simulink library "inp"  (Simulink 8.2)
settings: Solver=ode45  SolverMode=SingleTasking  StartTime=0.0  StopTime=10.0  RelTol=1e-3
undefined symbols (not defined in the file or in its folder): C_TICK_TIME_S, ENUM_FAULTSTATE_FAIL, ENUM_FAULTSTATE_INDETERMINATE, ENUM_FAULTSTATE_PASS, ENUM_KEYSWITCH_ON, FALSE, KINP_ABS1CANCommuOk_flg_ovrdflg, KINP_ABS1CANCommuOk_flg_ovrdval, KINP_ABS1CANPeriodTime_s, KINP_ABS1CANRolCntOk_flg_ovrdflg, KINP_ABS1CANRolCntOk_flg_ovrdval, KINP_ABS2CANChkSumOk_flg_ovrdflg, KINP_ABS2CANChkSumOk_flg_ovrdval, KINP_ABS2CANCommuOk_flg_ovrdflg, KINP_ABS2CANCommuOk_flg_ovrdval, KINP_ABS2CANPeriodTime_s, KINP_ABS2CANRolCntOk_flg_ovrdflg, KINP_ABS2CANRolCntOk_flg_ovrdval, KINP_ABS3CANCommuOk_flg_ovrdflg, KINP_ABS3CANCommuOk_flg_ovrdval, KINP_ABS4CANCommuOk_flg_ovrdflg, KINP_ABS4CANCommuOk_flg_ovrdval, KINP_ABSActive_flg_defval, KINP_ABSActive_flg_invalid, KINP_ABSActive_flg_ovrdflg, KINP_ABSActive_flg_ovrdval, KINP_ABSActive_flg_setvalid, KINP_ABSActive_flg_srvcflg, KINP_ABSActive_flg_srvcval, KINP_ABSCANAlive_flg_ovrdflg, KINP_ABSCANAlive_flg_ovrdval, KINP_ABSCANCommuOk_flg_ovrdflg, KINP_ABSCANCommuOk_flg_ovrdval, KINP_ABSCANValidOk_flg_ovrdflg, KINP_ABSCANValidOk_flg_ovrdval, KINP_ABSFault_flg_defval, KINP_ABSFault_flg_invalid, KINP_ABSFault_flg_ovrdflg, KINP_ABSFault_flg_ovrdval, KINP_ABSFault_flg_setvalid, KINP_ABSFault_flg_srvcflg, KINP_ABSFault_flg_srvcval, KINP_AC1CANCommuOk_flg_ovrdflg, KINP_AC1CANCommuOk_flg_ovrdval, KINP_ACCANAlive_flg_ovrdflg, KINP_ACCANAlive_flg_ovrdval, KINP_ACCANCommuOk_flg_ovrdflg, KINP_ACCANCommuOk_flg_ovrdval, KINP_ACCANValidOk_flg_ovrdflg, KINP_ACCANValidOk_flg_ovrdval, KINP_ACCCancelH_flg_defval, KINP_ACCCancelH_flg_invalid, KINP_ACCCancelH_flg_ovrdflg, KINP_ACCCancelH_flg_ovrdval, KINP_ACCCancelH_flg_setvalid, KINP_ACCCancelH_flg_srvcflg, KINP_ACCCancelH_flg_srvcval, KINP_AccelPedalPosn_pct_defval, KINP_AccelPedalPosn_pct_gain, KINP_AccelPedalPosn_pct_invalid ... (+1866)
libraries: inp x192, hcu_lib x34, simulink x33
(part 5 of 21)

== system inp/INP/METER_signals ==
  BHAL_outputs : Inport  OutDataTypeStr=Bus: BHAL_outputs
  CANvalid_bus : Inport  Port=2
  Bus Selector1 : BusSelector  OutputSignals=VHAL_MeterVehSpd_kph,VHAL_MeterVehSpdInvalid_flg
  Bus Selector10 : BusSelector  OutputSignals=VHAL_SeatBeltNOTFasten_flg
  Bus Selector12 : BusSelector  OutputSignals=VHAL_LowBrakeFluid_flg
  Bus Selector2 : BusSelector  OutputSignals=VINP_CANMsgValidMETER3_flg
  Bus Selector{4,6,9,22} (4 alike) : BusSelector  OutputSignals=VINP_CANMsgValidMETER1_flg
  Bus Selector5 : BusSelector  OutputSignals=VHAL_ParkBrakeOn_flg
  Bus Selector8 : BusSelector  OutputSignals=VHAL_OdoMeter_km,VHAL_OdoMeterInvalid_flg
  Data Store Read1 : DataStoreRead  DataStoreName=VDDML_METER3CANCommuFault_AF_flg
  Data Store Read{7..9,13} (4 alike) : DataStoreRead  DataStoreName=VDDML_METER1CANCommuFault_AF_flg
  Data Store Read14 : DataStoreRead  DataStoreName=VDDML_CANOdoMeterSigFault_AF_flg
  Data Store Read2 : DataStoreRead  DataStoreName=VDDML_CANMeterVehSpdSigFault_AF_flg
  Logical Operator1 : Logic  Tag=struct('inputs','1','comment',struct('text','','placement',1),'logdata',struct('maxnumvals',inf,'plotchannels',[],'stepsize',0,'downsampling',1,'loggingmode',3,'channels',1),'operator','NOT','output',struct('class','default','type','Bool8','description','','address','','unit','','name','$S_$B'),'date',731430.46749816)  Operator=NOT
  Logical Operator14 : Logic  Tag=struct('inputs','1','comment',struct('text','','placement',1),'logdata',struct('maxnumvals',inf,'plotchannels',[],'stepsize',0,'downsampling',1,'loggingmode',3,'channels',1),'operator','NOT','output',struct('class','default','type','Bool8','description','','address','','unit','','name','$S_$B'),'date',731430.46749816)  Operator=NOT
  Logical Operator2 : Logic  Tag=struct('inputs','1','comment',struct('text','','placement',1),'logdata',struct('maxnumvals',inf,'plotchannels',[],'stepsize',0,'downsampling',1,'loggingmode',3,'channels',1),'operator','NOT','output',struct('class','default','type','Bool8','description','','address','','unit','','name','$S_$B'),'date',731430.46749816)  Operator=OR
  Logical Operator6 : Logic  Tag=struct('inputs','1','comment',struct('text','','placement',1),'logdata',struct('maxnumvals',inf,'plotchannels',[],'stepsize',0,'downsampling',1,'loggingmode',3,'channels',1),'operator','NOT','output',struct('class','default','type','Bool8','description','','address','','unit','','name','$S_$B'),'date',731430.46749816)  Operator=OR
  LowBrakeFluid : Subsystem
      settings: RTWSystemCode=Inline
  MeterVehSpd : Subsystem
      settings: RTWSystemCode=Inline
  OdoMeter : Subsystem
      settings: RTWSystemCode=Inline
  ParkBrakeOn : Subsystem
      settings: RTWSystemCode=Inline
  SeatBeltNOTFasten : Subsystem
      settings: RTWSystemCode=Inline
  inputs_bus2 : BusCreator  Inputs=12
  METER_signal_bus : Outport
  wires:
  [BHAL_outputs] -> Bus Selector12/1
  LowBrakeFluid/2(signal_valid_out) -> inputs_bus2/5  "VINP_LowBrakeFluidValid_flg_InHAL"
  Bus Selector12/1 -> LowBrakeFluid/1(signal_in)  "<VHAL_LowBrakeFluid_flg>"
  LowBrakeFluid/1(signal_out) -> inputs_bus2/4  "VINP_LowBrakeFluid_flg_InHAL"
  Logical Operator6/1 -> OdoMeter/4(Active_fault_flg)
  Bus Selector9/1 -> OdoMeter/3(msg_valid)  "<VINP_CANMsgValidMETER1_flg>"
  [CANvalid_bus] -> Bus Selector9/1
  OdoMeter/3(raw_signal_valid_out) -> inputs_bus2/3  "VINP_OdoMeterSigOk_flg_InHAL"
  OdoMeter/2(signal_valid_out) -> inputs_bus2/2  "VINP_OdoMeterValid_flg_InHAL"
  OdoMeter/1(signal_out) -> inputs_bus2/1  "VINP_OdoMeter_km_InHAL"
  Data Store Read13/1 -> Logical Operator6/2
  Data Store Read14/1 -> Logical Operator6/1
  Logical Operator14/1 -> OdoMeter/2(signal_valid_in)
  Bus Selector8/2 -> Logical Operator14/1  "<VHAL_OdoMeterInvalid_flg>"
  Bus Selector8/1 -> OdoMeter/1(signal_in)  "<VHAL_OdoMeter_km>"
  [BHAL_outputs] -> Bus Selector8/1
  CANvalid_bus/1 -> [CANvalid_bus]
  BHAL_outputs/1 -> [BHAL_outputs]
  inputs_bus2/1 -> METER_signal_bus/1
  Bus Selector4/1 -> LowBrakeFluid/2(msg_valid)  "<VINP_CANMsgValidMETER1_flg>"
  [CANvalid_bus] -> Bus Selector4/1
  Data Store Read7/1 -> LowBrakeFluid/3(Active_fault_flg)
  [BHAL_outputs] -> Bus Selector5/1
  ParkBrakeOn/2(signal_valid_out) -> inputs_bus2/7  "VINP_ParkBrakeOnValid_flg_InHAL"
  Bus Selector5/1 -> ParkBrakeOn/1(signal_in)  "<VHAL_ParkBrakeOn_flg>"
  ParkBrakeOn/1(signal_out) -> inputs_bus2/6  "VINP_ParkBrakeOn_flg_InHAL"
  Bus Selector6/1 -> ParkBrakeOn/2(msg_valid)  "<VINP_CANMsgValidMETER1_flg>"
  [CANvalid_bus] -> Bus Selector6/1
  Data Store Read8/1 -> ParkBrakeOn/3(Active_fault_flg)
  [BHAL_outputs] -> Bus Selector10/1
  SeatBeltNOTFasten/2(signal_valid_out) -> inputs_bus2/9  "VINP_SeatBeltNOTFastenValid_flg_InHAL"
  Bus Selector10/1 -> SeatBeltNOTFasten/1(signal_in)  "<VHAL_SeatBeltNOTFasten_flg>"
  SeatBeltNOTFasten/1(signal_out) -> inputs_bus2/8  "VINP_SeatBeltNOTFasten_flg_InHAL"
  Bus Selector22/1 -> SeatBeltNOTFasten/2(msg_valid)  "<VINP_CANMsgValidMETER1_flg>"
  [CANvalid_bus] -> Bus Selector22/1
  Data Store Read9/1 -> SeatBeltNOTFasten/3(Active_fault_flg)
  Logical Operator2/1 -> MeterVehSpd/4(Active_fault_flg)
  Bus Selector2/1 -> MeterVehSpd/3(msg_valid)  "<VINP_CANMsgValidMETER3_flg>"
  [CANvalid_bus] -> Bus Selector2/1
  MeterVehSpd/3(raw_signal_valid_out) -> inputs_bus2/12  "VINP_MeterVehSpdSigOk_flg_InHAL"
  MeterVehSpd/2(signal_valid_out) -> inputs_bus2/11  "VINP_MeterVehSpdValid_flg_InHAL"
  MeterVehSpd/1(signal_out) -> inputs_bus2/10  "VINP_MeterVehSpd_kph_InHAL"
  Data Store Read1/1 -> Logical Operator2/2
  Data Store Read2/1 -> Logical Operator2/1
  Logical Operator1/1 -> MeterVehSpd/2(signal_valid_in)
  Bus Selector1/2 -> Logical Operator1/1  "<VHAL_MeterVehSpdInvalid_flg>"
  Bus Selector1/1 -> MeterVehSpd/1(signal_in)  "<VHAL_MeterVehSpd_kph>"
  [BHAL_outputs] -> Bus Selector1/1

== system inp/INP/TCM_signals ==
  BHAL_outputs : Inport  OutDataTypeStr=Bus: BHAL_outputs
  CANvalid_bus : Inport  Port=2
  Bus Selector{1..2,5,10..11,13,16,31} (8 alike) : BusSelector  OutputSignals=VINP_CANMsgValidTCM1_flg
  Bus Selector12 : BusSelector  OutputSignals=VHAL_TCMMILReq_flg
  Bus Selector14 : BusSelector  OutputSignals=VHAL_TCMFaultStatus_enum
  Bus Selector15 : BusSelector  OutputSignals=VHAL_TCMWinterMode_flg
  Bus Selector17 : BusSelector  OutputSignals=VHAL_TCMPowerMode_flg
  Bus Selector3 : BusSelector  OutputSignals=VHAL_TMTurbineSpd_rpm,VHAL_TMTurbineSpdInvalid_flg
  Bus Selector4 : BusSelector  OutputSignals=VHAL_ShifterPosn_enum,VHAL_ShifterPosnInvalid_flg
  Bus Selector6 : BusSelector  OutputSignals=VHAL_NowGearPosn_enum,VHAL_NowGearPosnInvalid_flg
  Bus Selector7 : BusSelector  OutputSignals=VHAL_DriverMode_enum
  Bus Selector8 : BusSelector  OutputSignals=VINP_CANMsgValidTCM2_flg
  Bus Selector9 : BusSelector  OutputSignals=VHAL_TqConvClutchSt_enum
  Constant{,1..3} (4 alike) : Constant  Value=TRUE
  Data Store Read{1..4,20..21,23,25} (8 alike) : DataStoreRead  DataStoreName=VDDML_TCM1CANCommuFault_AF_flg
  Data Store Read18 : DataStoreRead  DataStoreName=VDDML_CANNowGearPosnSigFault_AF_flg
  Data Store Read19 : DataStoreRead  DataStoreName=VDDML_CANShftPosnSigFault_AF_flg
  Data Store Read24 : DataStoreRead  DataStoreName=VDDML_CANTMTurbSpdSigFault_AF_flg
  Data Store Read32 : DataStoreRead  DataStoreName=VDDML_TCM2CANCommuFault_AF_flg
  Data Type Conversion : DataTypeConversion  OutDataTypeStr=uint8
  Data Type Conversion1 : DataTypeConversion  OutDataTypeStr=boolean
  Data Type Conversion2 : DataTypeConversion  OutDataTypeStr=boolean
  Data Type Conversion3 : DataTypeConversion  OutDataTypeStr=boolean
  DriverMode : Subsystem
      settings: RTWSystemCode=Inline
  Logical Operator1 : Logic  Tag=struct('inputs','1','comment',struct('text','','placement',1),'logdata',struct('maxnumvals',inf,'plotchannels',[],'stepsize',0,'downsampling',1,'loggingmode',3,'channels',1),'operator','NOT','output',struct('class','default','type','Bool8','description','','address','','unit','','name','$S_$B'),'date',731430.46749816)  Operator=OR
  Logical Operator2 : Logic  Tag=struct('inputs','1','comment',struct('text','','placement',1),'logdata',struct('maxnumvals',inf,'plotchannels',[],'stepsize',0,'downsampling',1,'loggingmode',3,'channels',1),'operator','NOT','output',struct('class','default','type','Bool8','description','','address','','unit','','name','$S_$B'),'date',731430.46749816)  Operator=OR
  Logical Operator3 : Logic  Tag=struct('inputs','1','comment',struct('text','','placement',1),'logdata',struct('maxnumvals',inf,'plotchannels',[],'stepsize',0,'downsampling',1,'loggingmode',3,'channels',1),'operator','NOT','output',struct('class','default','type','Bool8','description','','address','','unit','','name','$S_$B'),'date',731430.46749816)  Operator=NOT  Inputs=1
  Logical Operator4 : Logic  Tag=struct('inputs','1','comment',struct('text','','placement',1),'logdata',struct('maxnumvals',inf,'plotchannels',[],'stepsize',0,'downsampling',1,'loggingmode',3,'channels',1),'operator','NOT','output',struct('class','default','type','Bool8','description','','address','','unit','','name','$S_$B'),'date',731430.46749816)  Operator=OR
  Logical Operator5 : Logic  Tag=struct('inputs','1','comment',struct('text','','placement',1),'logdata',struct('maxnumvals',inf,'plotchannels',[],'stepsize',0,'downsampling',1,'loggingmode',3,'channels',1),'operator','NOT','output',struct('class','default','type','Bool8','description','','address','','unit','','name','$S_$B'),'date',731430.46749816)  Operator=NOT  Inputs=1
  Logical Operator8 : Logic  Tag=struct('inputs','1','comment',struct('text','','placement',1),'logdata',struct('maxnumvals',inf,'plotchannels',[],'stepsize',0,'downsampling',1,'loggingmode',3,'channels',1),'operator','NOT','output',struct('class','default','type','Bool8','description','','address','','unit','','name','$S_$B'),'date',731430.46749816)  Operator=NOT  Inputs=1
  NowGearPosn : Subsystem
      settings: RTWSystemCode=Inline
  ShifterPosn : Subsystem
      settings: RTWSystemCode=Inline
  TCMFaultStatus : Subsystem
      settings: RTWSystemCode=Inline
  TMTurbineSpd : Subsystem
      settings: RTWSystemCode=Inline
  Terminator{,1..7} (8 alike) : Terminator
  inputs_bus2 : BusCreator  Inputs=21
  TCM_signal_bus : Outport
  wires:
  inputs_bus2/1 -> TCM_signal_bus/1
  BHAL_outputs/1 -> [BHAL_outputs]
  CANvalid_bus/1 -> [CANvalid_bus]
  Logical Operator8/1 -> ShifterPosn/2(signal_valid_in)
  Bus Selector4/1 -> ShifterPosn/1(signal_in)  "<VHAL_ShifterPosn_enum>"
  [BHAL_outputs] -> Bus Selector4/1
  ShifterPosn/2(signal_valid_out) -> inputs_bus2/2  "VINP_ShifterPosnValid_flg_InHAL"
  ShifterPosn/1(signal_out) -> inputs_bus2/1  "VINP_ShifterPosn_enum_InHAL"
  Bus Selector4/2 -> Logical Operator8/1  "<VHAL_ShifterPosnInvalid_flg>"
  Data Store Read19/1 -> Logical Operator2/1
  [CANvalid_bus] -> Bus Selector1/1
  Bus Selector1/1 -> ShifterPosn/3(msg_valid)  "<VINP_CANMsgValidTCM1_flg>"
  Logical Operator2/1 -> ShifterPosn/4(Active_fault_flg)
  Data Store Read20/1 -> Logical Operator2/2
  ShifterPosn/3(raw_signal_valid_out) -> inputs_bus2/3  "VINP_ShifterPosnSigOk_flg_InHAL"
  Logical Operator3/1 -> NowGearPosn/2(signal_valid_in)
  Bus Selector6/1 -> NowGearPosn/1(signal_in)  "<VHAL_NowGearPosn_enum>"
  [BHAL_outputs] -> Bus Selector6/1
  NowGearPosn/2(signal_valid_out) -> inputs_bus2/5  "VINP_NowGearPosnValid_flg_InHAL"
  NowGearPosn/1(signal_out) -> inputs_bus2/4  "VINP_NowGearPosn_enum_InHAL"
  Bus Selector6/2 -> Logical Operator3/1  "<VHAL_NowGearPosnInvalid_flg>"
  Data Store Read18/1 -> Logical Operator1/1
  [CANvalid_bus] -> Bus Selector5/1
  Bus Selector5/1 -> NowGearPosn/3(msg_valid)  "<VINP_CANMsgValidTCM1_flg>"
  Logical Operator1/1 -> NowGearPosn/4(Active_fault_flg)
  Data Store Read21/1 -> Logical Operator1/2
  NowGearPosn/3(raw_signal_valid_out) -> inputs_bus2/6  "VINP_NowGearPosnSigOk_flg_InHAL"
  TMTurbineSpd/1(signal_out) -> inputs_bus2/7  "VINP_TMTurbineSpd_rpm_InHAL"
  TMTurbineSpd/2(signal_valid_out) -> inputs_bus2/8  "VINP_TMTurbineSpdValid_flg_InHAL"
  TMTurbineSpd/3(raw_signal_valid_out) -> inputs_bus2/9  "VINP_TMTurbineSpdSigOk_flg_InHAL"
  Logical Operator5/1 -> TMTurbineSpd/2(signal_valid_in)
  Bus Selector3/1 -> TMTurbineSpd/1(signal_in)  "<VHAL_TMTurbineSpd_rpm>"
  [BHAL_outputs] -> Bus Selector3/1
  Bus Selector3/2 -> Logical Operator5/1  "<VHAL_TMTurbineSpdInvalid_flg>"
  Data Store Read24/1 -> Logical Operator4/1
  [CANvalid_bus] -> Bus Selector2/1
  Bus Selector2/1 -> TMTurbineSpd/3(msg_valid)  "<VINP_CANMsgValidTCM1_flg>"
  Logical Operator4/1 -> TMTurbineSpd/4(Active_fault_flg)
  Data Store Read25/1 -> Logical Operator4/2
  Bus Selector14/1 -> TCMFaultStatus/1(signal_in)  "<VHAL_TCMFaultStatus_enum>"
  [BHAL_outputs] -> Bus Selector14/1
  TCMFaultStatus/1(signal_out) -> inputs_bus2/10  "VINP_TCMFaultStatus_enum_InHAL"
  TCMFaultStatus/2(signal_valid_out) -> inputs_bus2/11  "VINP_TCMFaultStatusValid_flg_InHAL"
  Bus Selector31/1 -> TCMFaultStatus/2(msg_valid)  "<VINP_CANMsgValidTCM1_flg>"
  [CANvalid_bus] -> Bus Selector31/1
  Data Store Read23/1 -> TCMFaultStatus/3(Active_fault_flg)
  Bus Selector7/1 -> DriverMode/1(signal_in)  "<VHAL_DriverMode_enum>"
  [BHAL_outputs] -> Bus Selector7/1
  DriverMode/1(signal_out) -> inputs_bus2/20  "VINP_DriverMode_enum_InHAL"
  DriverMode/2(signal_valid_out) -> inputs_bus2/21  "VINP_DriverModeValid_flg_InHAL"
  Bus Selector8/1 -> DriverMode/2(msg_valid)  "<VINP_CANMsgValidTCM2_flg>"
  [CANvalid_bus] -> Bus Selector8/1
  Data Store Read32/1 -> DriverMode/3(Active_fault_flg)
  Bus Selector9/1 -> Data Type Conversion/1  "<VHAL_TqConvClutchSt_enum>"
  [BHAL_outputs] -> Bus Selector9/1
  Data Type Conversion/1 -> inputs_bus2/12  "VINP_TqConvClutchSt_enum_InHAL"
  Constant/1 -> inputs_bus2/13  "VINP_TqConvClutchStValid_flg_InHAL"
  Data Store Read2/1 -> Terminator1/1
  Bus Selector10/1 -> Terminator/1  "<VINP_CANMsgValidTCM1_flg>"
  [CANvalid_bus] -> Bus Selector10/1
  Bus Selector12/1 -> Data Type Conversion1/1  "<VHAL_TCMMILReq_flg>"
  [BHAL_outputs] -> Bus Selector12/1
  Data Type Conversion1/1 -> inputs_bus2/14  "VINP_TCMMILReq_flg_InHAL"
  Constant1/1 -> inputs_bus2/15  "VINP_TCMMILReqValid_flg_InHAL"
  Data Store Read1/1 -> Terminator3/1
  Bus Selector11/1 -> Terminator2/1  "<VINP_CANMsgValidTCM1_flg>"
  [CANvalid_bus] -> Bus Selector11/1
  Bus Selector15/1 -> Data Type Conversion2/1  "<VHAL_TCMWinterMode_flg>"
  [BHAL_outputs] -> Bus Selector15/1
  Data Type Conversion2/1 -> inputs_bus2/16  "VINP_TCMWinterMode_flg_InHAL"
  Constant2/1 -> inputs_bus2/17  "VINP_TCMWinterModeValid_flg_InHAL"
  Data Store Read3/1 -> Terminator5/1
  Bus Selector13/1 -> Terminator4/1  "<VINP_CANMsgValidTCM1_flg>"
  [CANvalid_bus] -> Bus Selector13/1
  Bus Selector17/1 -> Data Type Conversion3/1  "<VHAL_TCMPowerMode_flg>"
  [BHAL_outputs] -> Bus Selector17/1
  Data Type Conversion3/1 -> inputs_bus2/18  "VINP_TCMPowerMode_flg_InHAL"
  Constant3/1 -> inputs_bus2/19  "VINP_TCMPowerModeValid_flg_InHAL"
  Data Store Read4/1 -> Terminator7/1
  Bus Selector16/1 -> Terminator6/1  "<VINP_CANMsgValidTCM1_flg>"
  [CANvalid_bus] -> Bus Selector16/1

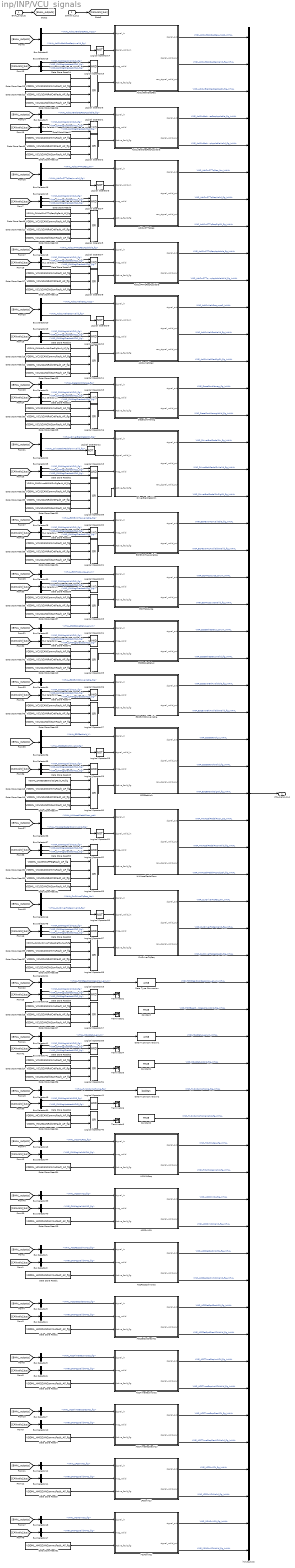
== system inp/INP/VCU_signals ==
  BHAL_outputs : Inport  OutDataTypeStr=Bus: BHAL_outputs
  CANvalid_bus : Inport  Port=2
  ACCOnVCU : Subsystem
      settings: RTWSystemCode=Inline
  ACCResSpdIncVCU : Subsystem
      settings: RTWSystemCode=Inline
  ACCSetSpdDecVCU : Subsystem
      settings: RTWSystemCode=Inline
  ACCTimeGapDecVCU : Subsystem
      settings: RTWSystemCode=Inline
  ACCTimeGapIncVCU : Subsystem
      settings: RTWSystemCode=Inline
  AckCurBrakDecReq : Subsystem
      settings: RTWSystemCode=Inline
  AckCurBrakDecReqApplable : Subsystem
      settings: RTWSystemCode=Inline
  AckCurPTTqReq : Subsystem
      settings: RTWSystemCode=Inline
  AckCurPTTqReqApplable : Subsystem
      settings: RTWSystemCode=Inline
  AckCurVehDec : Subsystem
      settings: RTWSystemCode=Inline
  Bus Selector1 : BusSelector  OutputSignals=VHAL_ACCResSpdIncVCU_flg
  Bus Selector{2,4,6,10..11,40,47} (7 alike) : BusSelector  OutputSignals=VINP_CANMsgValidHMI2_flg
  Bus Selector12 : BusSelector  OutputSignals=VHAL_LFCOnVCU_flg
  Bus Selector{13..15,17,19,23,25,27,29,31,33,35} (12 alike) : BusSelector  OutputSignals=VINP_CANMsgValidVCU1_flg,VINP_CANMsgUpdatedVCU1_flg,VINP_CANMsgCheckedVCU1_flg
  Bus Selector16 : BusSelector  OutputSignals=VHAL_AckCurPTTqReq_Nm,VHAL_AckCurPTTqReqInvalid_flg
  Bus Selector18 : BusSelector  OutputSignals=VHAL_AckCurPTTqReqApplable_flg
  Bus Selector20 : BusSelector  OutputSignals=VHAL_AckCurVehDec_mps2,VHAL_AckCurVehDecInvalid_flg
  Bus Selector21 : BusSelector  OutputSignals=VHAL_TCMGearPosnReqAllow_enum
  Bus Selector22 : BusSelector  OutputSignals=VHAL_DriverBrakPedalOn_flg,VHAL_DriverBrakPedalOnInvalid_flg
  Bus Selector24 : BusSelector  OutputSignals=VHAL_DeceContManag_flg
  Bus Selector{26,37,42,45} (4 alike) : BusSelector  OutputSignals=VINP_CANMsgValidVCU2_flg,VINP_CANMsgUpdatedVCU2_flg,VINP_CANMsgCheckedVCU2_flg
  Bus Selector28 : BusSelector  OutputSignals=VHAL_ECMExtnTqAvailable_flg
  Bus Selector3 : BusSelector  OutputSignals=VHAL_ACCSetSpdDecVCU_flg
  Bus Selector30 : BusSelector  OutputSignals=VHAL_ECMTqSource_enum
  Bus Selector32 : BusSelector  OutputSignals=VHAL_ESCBrakeStatus_enum
  Bus Selector34 : BusSelector  OutputSignals=VHAL_ESCExtnBrkAvailable_flg
  Bus Selector36 : BusSelector  OutputSignals=VHAL_ESCBattVolt_V,VHAL_ESCBattVoltInvalid_flg
  Bus Selector38 : BusSelector  OutputSignals=VHAL_HWAccelPedalPosn_pct,VHAL_HWAccelPedalPosnInvalid_flg
  Bus Selector39 : BusSelector  OutputSignals=VHAL_ACCOnVCU_flg
  Bus Selector41 : BusSelector  OutputSignals=VHAL_MSAStatus_enum
  Bus Selector43 : BusSelector  OutputSignals=VHAL_HCUMILReq_flg
  Bus Selector44 : BusSelector  OutputSignals=VINP_CANMsgValidHCU4_flg
  Bus Selector46 : BusSelector  OutputSignals=VHAL_CurDriverTqReq_Nm,VHAL_CurDriverTqReqInvalid_flg
  Bus Selector48 : BusSelector  OutputSignals=VHAL_MSAOnVCU_flg
  Bus Selector49 : BusSelector  OutputSignals=VHAL_HybridLimpHome_flg
  Bus Selector5 : BusSelector  OutputSignals=VHAL_ACCTimeGapIncVCU_flg
  Bus Selector50 : BusSelector  OutputSignals=VINP_CANMsgValidHCU3_flg,VINP_CANMsgUpdatedHCU3_flg
  Bus Selector7 : BusSelector  OutputSignals=VHAL_ACCTimeGapDecVCU_flg
  Bus Selector8 : BusSelector  OutputSignals=VHAL_AckCurBrakDecReq_mps2,VHAL_AckCurBrakDecReqInvalid_flg
  Bus Selector9 : BusSelector  OutputSignals=VHAL_AckCurBrakDecReqApplable_flg
  Constant : Constant  Value=TRUE
  Constant1 : Constant  Value=TRUE
  Constant2 : Constant  Value=TRUE
  CurDriverTqReq : Subsystem
      settings: RTWSystemCode=Inline
  Data Store Read{1..5,19,61} (7 alike) : DataStoreRead  DataStoreName=VDDML_HMI2CANCommuFault_AF_flg
  Data Store Read{10,12,15,23,26,28,31,34,37,40,43,47} (12 alike) : DataStoreRead  DataStoreName=VDDML_VCU1CANChkSumFault_AF_flg
  Data Store Read{9,11,17,21..22,30,33,36,39,42,45..46} (12 alike) : DataStoreRead  DataStoreName=VDDML_VCU1CANRolCntFault_AF_flg
  Data Store Read{6..7,13,16,18,29,32,35,38,41,44,48} (12 alike) : DataStoreRead  DataStoreName=VDDML_VCU1CANCommuFault_AF_flg
  Data Store Read14 : DataStoreRead  DataStoreName=VDDML_CANAckCurBrakDecReqSigFault_AF_flg
  Data Store Read20 : DataStoreRead  DataStoreName=VDDML_CANAckCurVehDecSigFault_AF_flg
  Data Store Read{24..25,50,57} (4 alike) : DataStoreRead  DataStoreName=VDDML_VCU2CANRolCntFault_AF_flg
  Data Store Read{27,51,56,58} (4 alike) : DataStoreRead  DataStoreName=VDDML_VCU2CANChkSumFault_AF_flg
  Data Store Read49 : DataStoreRead  DataStoreName=VDDML_CANESCBattVoltSigFault_AF_flg
  Data Store Read{52,55,59,63} (4 alike) : DataStoreRead  DataStoreName=VDDML_VCU2CANCommuFault_AF_flg
  Data Store Read53 : DataStoreRead  DataStoreName=VDDML_CANHWAPPSigFault_AF_flg
  Data Store Read54 : DataStoreRead  DataStoreName=VDDML_HCU4CANCommuFault_AF_flg
  Data Store Read60 : DataStoreRead  DataStoreName=VDDML_CANCurDriverTqReqSigFault_AF_flg
  Data Store Read62 : DataStoreRead  DataStoreName=VDDML_CANDriverBrkPdlOnSigFault_AF_flg
  Data Store Read64 : DataStoreRead  DataStoreName=VDDML_HCU3CANRolCntFault_AF_flg
  Data Store Read66 : DataStoreRead  DataStoreName=VDDML_HCU3CANCommuFault_AF_flg
  Data Store Read8 : DataStoreRead  DataStoreName=VDDML_CANAckCurPTTqReqSigFault_AF_flg
  Data Type Conversion : DataTypeConversion  OutDataTypeStr=uint8
  Data Type Conversion1 : DataTypeConversion  OutDataTypeStr=uint8
  Data Type Conversion2 : DataTypeConversion  OutDataTypeStr=boolean
  DeceContManag : Subsystem
      settings: RTWSystemCode=Inline
  DriverBrakPedalOn : Subsystem
      settings: RTWSystemCode=Inline
  ECMExtnTqAvailable : Subsystem
      settings: RTWSystemCode=Inline
  ECMTqSource : Subsystem
      settings: RTWSystemCode=Inline
  ESCBattVolt : Subsystem
      settings: RTWSystemCode=Inline
  ESCBrakeStatus : Subsystem
      settings: RTWSystemCode=Inline
  ESCExtnBrkAvailable : Subsystem
      settings: RTWSystemCode=Inline
  HCUMILReq : Subsystem
      settings: RTWSystemCode=Inline
  HWAccelPedalPosn : Subsystem
      settings: RTWSystemCode=Inline
  LFCOnVCU : Subsystem
      settings: RTWSystemCode=Inline
  Logical Operator{1,4,7,10,13,15,18..20,22,24,26,29,32,35,37} (16 alike) : Logic  Tag=struct('inputs','1','comment',struct('text','','placement',1),'logdata',struct('maxnumvals',inf,'plotchannels',[],'stepsize',0,'downsampling',1,'loggingmode',3,'channels',1),'operator','NOT','output',struct('class','default','type','Bool8','description','','address','','unit','','name','$S_$B'),'date',731430.46749816)  Inputs=3
  Logical Operator{5..6,11,30,33,38..39} (7 alike) : Logic  Tag=struct('inputs','1','comment',struct('text','','placement',1),'logdata',struct('maxnumvals',inf,'plotchannels',[],'stepsize',0,'downsampling',1,'loggingmode',3,'channels',1),'operator','NOT','output',struct('class','default','type','Bool8','description','','address','','unit','','name','$S_$B'),'date',731430.46749816)  Operator=OR  Inputs=4
  Logical Operator12 : Logic  Tag=struct('inputs','1','comment',struct('text','','placement',1),'logdata',struct('maxnumvals',inf,'plotchannels',[],'stepsize',0,'downsampling',1,'loggingmode',3,'channels',1),'operator','NOT','output',struct('class','default','type','Bool8','description','','address','','unit','','name','$S_$B'),'date',731430.46749816)  Operator=NOT  Inputs=1
  Logical Operator{3,9,14,28,31,36} (6 alike) : Logic  Tag=struct('inputs','1','comment',struct('text','','placement',1),'logdata',struct('maxnumvals',inf,'plotchannels',[],'stepsize',0,'downsampling',1,'loggingmode',3,'channels',1),'operator','NOT','output',struct('class','default','type','Bool8','description','','address','','unit','','name','$S_$B'),'date',731430.46749816)  Operator=NOT
  Logical Operator{2,8,16..17,21,23,25,27,34} (9 alike) : Logic  Tag=struct('inputs','1','comment',struct('text','','placement',1),'logdata',struct('maxnumvals',inf,'plotchannels',[],'stepsize',0,'downsampling',1,'loggingmode',3,'channels',1),'operator','NOT','output',struct('class','default','type','Bool8','description','','address','','unit','','name','$S_$B'),'date',731430.46749816)  Operator=OR  Inputs=3
  Logical Operator40 : Logic  Tag=struct('inputs','1','comment',struct('text','','placement',1),'logdata',struct('maxnumvals',inf,'plotchannels',[],'stepsize',0,'downsampling',1,'loggingmode',3,'channels',1),'operator','NOT','output',struct('class','default','type','Bool8','description','','address','','unit','','name','$S_$B'),'date',731430.46749816)  Operator=OR
  Logical Operator41 : Logic  Tag=struct('inputs','1','comment',struct('text','','placement',1),'logdata',struct('maxnumvals',inf,'plotchannels',[],'stepsize',0,'downsampling',1,'loggingmode',3,'channels',1),'operator','NOT','output',struct('class','default','type','Bool8','description','','address','','unit','','name','$S_$B'),'date',731430.46749816)
  MSAOnVCU : Subsystem
      settings: RTWSystemCode=Inline
  Terminator{1..6} (6 alike) : Terminator
  inputs_bus2 : BusCreator  Inputs=57
  VCU_signal_bus : Outport
  wires:
  inputs_bus2/1 -> VCU_signal_bus/1
  BHAL_outputs/1 -> [BHAL_outputs]
  CANvalid_bus/1 -> [CANvalid_bus]
  Bus Selector40/1 -> ACCOnVCU/2(msg_valid)  "<VINP_CANMsgValidHMI2_flg>"
  [CANvalid_bus] -> Bus Selector40/1
  Bus Selector39/1 -> ACCOnVCU/1(signal_in)  "<VHAL_ACCOnVCU_flg>"
  [BHAL_outputs] -> Bus Selector39/1
  ACCOnVCU/1(signal_out) -> inputs_bus2/44  "VINP_ACCOnVCU_flg_InHAL"
  ACCOnVCU/2(signal_valid_out) -> inputs_bus2/45  "VINP_ACCOnVCUValid_flg_InHAL"
  Data Store Read19/1 -> ACCOnVCU/3(Active_fault_flg)
  Bus Selector2/1 -> ACCResSpdIncVCU/2(msg_valid)  "<VINP_CANMsgValidHMI2_flg>"
  [CANvalid_bus] -> Bus Selector2/1
  Bus Selector1/1 -> ACCResSpdIncVCU/1(signal_in)  "<VHAL_ACCResSpdIncVCU_flg>"
  [BHAL_outputs] -> Bus Selector1/1
  ACCResSpdIncVCU/1(signal_out) -> inputs_bus2/46  "VINP_ACCResSpdIncVCU_flg_InHAL"
  ACCResSpdIncVCU/2(signal_valid_out) -> inputs_bus2/47  "VINP_ACCResSpdIncVCUValid_flg_InHAL"
  Data Store Read1/1 -> ACCResSpdIncVCU/3(Active_fault_flg)
  Bus Selector4/1 -> ACCSetSpdDecVCU/2(msg_valid)  "<VINP_CANMsgValidHMI2_flg>"
  [CANvalid_bus] -> Bus Selector4/1
  Bus Selector3/1 -> ACCSetSpdDecVCU/1(signal_in)  "<VHAL_ACCSetSpdDecVCU_flg>"
  [BHAL_outputs] -> Bus Selector3/1
  ACCSetSpdDecVCU/1(signal_out) -> inputs_bus2/48  "VINP_ACCSetSpdDecVCU_flg_InHAL"
  ACCSetSpdDecVCU/2(signal_valid_out) -> inputs_bus2/49  "VINP_ACCSetSpdDecVCUValid_flg_InHAL"
  Data Store Read2/1 -> ACCSetSpdDecVCU/3(Active_fault_flg)
  Bus Selector6/1 -> ACCTimeGapIncVCU/2(msg_valid)  "<VINP_CANMsgValidHMI2_flg>"
  [CANvalid_bus] -> Bus Selector6/1
  Bus Selector5/1 -> ACCTimeGapIncVCU/1(signal_in)  "<VHAL_ACCTimeGapIncVCU_flg>"
  [BHAL_outputs] -> Bus Selector5/1
  ACCTimeGapIncVCU/1(signal_out) -> inputs_bus2/50  "VINP_ACCTimeGapIncVCU_flg_InHAL"
  ACCTimeGapIncVCU/2(signal_valid_out) -> inputs_bus2/51  "VINP_ACCTimeGapIncVCUValid_flg_InHAL"
  Data Store Read3/1 -> ACCTimeGapIncVCU/3(Active_fault_flg)
  Bus Selector10/1 -> ACCTimeGapDecVCU/2(msg_valid)  "<VINP_CANMsgValidHMI2_flg>"
  [CANvalid_bus] -> Bus Selector10/1
  Bus Selector7/1 -> ACCTimeGapDecVCU/1(signal_in)  "<VHAL_ACCTimeGapDecVCU_flg>"
  [BHAL_outputs] -> Bus Selector7/1
  ACCTimeGapDecVCU/1(signal_out) -> inputs_bus2/52  "VINP_ACCTimeGapDecVCU_flg_InHAL"
  ACCTimeGapDecVCU/2(signal_valid_out) -> inputs_bus2/53  "VINP_ACCTimeGapDecVCUValid_flg_InHAL"
  Data Store Read4/1 -> ACCTimeGapDecVCU/3(Active_fault_flg)
  Bus Selector11/1 -> LFCOnVCU/2(msg_valid)  "<VINP_CANMsgValidHMI2_flg>"
  [CANvalid_bus] -> Bus Selector11/1
  Bus Selector12/1 -> LFCOnVCU/1(signal_in)  "<VHAL_LFCOnVCU_flg>"
  [BHAL_outputs] -> Bus Selector12/1
  LFCOnVCU/1(signal_out) -> inputs_bus2/54  "VINP_LFCOnVCU_flg_InHAL"
  LFCOnVCU/2(signal_valid_out) -> inputs_bus2/55  "VINP_LFCOnVCUValid_flg_InHAL"
  Data Store Read5/1 -> LFCOnVCU/3(Active_fault_flg)
  [BHAL_outputs] -> Bus Selector8/1
  Bus Selector8/1 -> AckCurBrakDecReq/1(signal_in)  "<VHAL_AckCurBrakDecReq_mps2>"
  Bus Selector8/2 -> Logical Operator14/1  "<VHAL_AckCurBrakDecReqInvalid_flg>"
  Logical Operator14/1 -> AckCurBrakDecReq/2(signal_valid_in)
  Data Store Read14/1 -> Logical Operator6/1
  Data Store Read13/1 -> Logical Operator6/2
  AckCurBrakDecReq/1(signal_out) -> inputs_bus2/1  "VINP_AckCurBrakDecReq_mps2_InHAL"
  AckCurBrakDecReq/2(signal_valid_out) -> inputs_bus2/2  "VINP_AckCurBrakDecReqValid_flg_InHAL"
  AckCurBrakDecReq/3(raw_signal_valid_out) -> inputs_bus2/3  "VINP_AckCurBrakDecReqSigOk_flg_InHAL"
  Logical Operator6/1 -> AckCurBrakDecReq/4(Active_fault_flg)
  Data Store Read21/1 -> Logical Operator6/3
  Data Store Read26/1 -> Logical Operator6/4
  [CANvalid_bus] -> Bus Selector15/1
  Bus Selector15/1 -> Logical Operator19/1  "<VINP_CANMsgValidVCU1_flg>"
  Bus Selector15/2 -> Logical Operator19/2  "<VINP_CANMsgUpdatedVCU1_flg>"
  Bus Selector15/3 -> Logical Operator19/3  "<VINP_CANMsgCheckedVCU1_flg>"
  Logical Operator19/1 -> AckCurBrakDecReq/3(msg_valid)
  Bus Selector9/1 -> AckCurBrakDecReqApplable/1(signal_in)  "<VHAL_AckCurBrakDecReqApplable_flg>"
  [BHAL_outputs] -> Bus Selector9/1
  AckCurBrakDecReqApplable/1(signal_out) -> inputs_bus2/4  "VINP_AckCurBrakDecReqApplable_flg_InHAL"
  AckCurBrakDecReqApplable/2(signal_valid_out) -> inputs_bus2/5  "VINP_AckCurBrakDecReqApplableValid_flg_InHAL"
  Data Store Read7/1 -> Logical Operator2/1
  Logical Operator2/1 -> AckCurBrakDecReqApplable/3(Active_fault_flg)
  Data Store Read9/1 -> Logical Operator2/2
  Data Store Read10/1 -> Logical Operator2/3
  [CANvalid_bus] -> Bus Selector14/1
  Bus Selector14/1 -> Logical Operator1/1  "<VINP_CANMsgValidVCU1_flg>"
  Bus Selector14/2 -> Logical Operator1/2  "<VINP_CANMsgUpdatedVCU1_flg>"
  Bus Selector14/3 -> Logical Operator1/3  "<VINP_CANMsgCheckedVCU1_flg>"
  Logical Operator1/1 -> AckCurBrakDecReqApplable/2(msg_valid)
  [BHAL_outputs] -> Bus Selector16/1
  Bus Selector16/1 -> AckCurPTTqReq/1(signal_in)  "<VHAL_AckCurPTTqReq_Nm>"
  Bus Selector16/2 -> Logical Operator3/1  "<VHAL_AckCurPTTqReqInvalid_flg>"
  Logical Operator3/1 -> AckCurPTTqReq/2(signal_valid_in)
  Data Store Read8/1 -> Logical Operator5/1
  Data Store Read6/1 -> Logical Operator5/2
  AckCurPTTqReq/1(signal_out) -> inputs_bus2/6  "VINP_AckCurPTTqReq_Nm_InHAL"
  AckCurPTTqReq/2(signal_valid_out) -> inputs_bus2/7  "VINP_AckCurPTTqReqValid_flg_InHAL"
  AckCurPTTqReq/3(raw_signal_valid_out) -> inputs_bus2/8  "VINP_AckCurPTTqReqSigOk_flg_InHAL"
  Logical Operator5/1 -> AckCurPTTqReq/4(Active_fault_flg)
  Data Store Read11/1 -> Logical Operator5/3
  Data Store Read12/1 -> Logical Operator5/4
  [CANvalid_bus] -> Bus Selector13/1
  Bus Selector13/1 -> Logical Operator4/1  "<VINP_CANMsgValidVCU1_flg>"
  Bus Selector13/2 -> Logical Operator4/2  "<VINP_CANMsgUpdatedVCU1_flg>"
  Bus Selector13/3 -> Logical Operator4/3  "<VINP_CANMsgCheckedVCU1_flg>"
  Logical Operator4/1 -> AckCurPTTqReq/3(msg_valid)
  Bus Selector18/1 -> AckCurPTTqReqApplable/1(signal_in)  "<VHAL_AckCurPTTqReqApplable_flg>"
  [BHAL_outputs] -> Bus Selector18/1
  AckCurPTTqReqApplable/1(signal_out) -> inputs_bus2/9  "VINP_AckCurPTTqReqApplable_flg_InHAL"
  AckCurPTTqReqApplable/2(signal_valid_out) -> inputs_bus2/10  "VINP_AckCurPTTqReqApplableValid_flg_InHAL"
  Data Store Read16/1 -> Logical Operator8/1
  Logical Operator8/1 -> AckCurPTTqReqApplable/3(Active_fault_flg)
  Data Store Read17/1 -> Logical Operator8/2
  Data Store Read15/1 -> Logical Operator8/3
  [CANvalid_bus] -> Bus Selector17/1
  Bus Selector17/1 -> Logical Operator7/1  "<VINP_CANMsgValidVCU1_flg>"
  Bus Selector17/2 -> Logical Operator7/2  "<VINP_CANMsgUpdatedVCU1_flg>"
  Bus Selector17/3 -> Logical Operator7/3  "<VINP_CANMsgCheckedVCU1_flg>"
  Logical Operator7/1 -> AckCurPTTqReqApplable/2(msg_valid)
  [BHAL_outputs] -> Bus Selector20/1
  Bus Selector20/1 -> AckCurVehDec/1(signal_in)  "<VHAL_AckCurVehDec_mps2>"
  Bus Selector20/2 -> Logical Operator9/1  "<VHAL_AckCurVehDecInvalid_flg>"
  Logical Operator9/1 -> AckCurVehDec/2(signal_valid_in)
  Data Store Read20/1 -> Logical Operator11/1
  Data Store Read18/1 -> Logical Operator11/2
  AckCurVehDec/1(signal_out) -> inputs_bus2/11  "VINP_AckCurVehDec_mps2_InHAL"
  AckCurVehDec/2(signal_valid_out) -> inputs_bus2/12  "VINP_AckCurVehDecValid_flg_InHAL"
  AckCurVehDec/3(raw_signal_valid_out) -> inputs_bus2/13  "VINP_AckCurVehDecSigOk_flg_InHAL"
  Logical Operator11/1 -> AckCurVehDec/4(Active_fault_flg)
  Data Store Read22/1 -> Logical Operator11/3
  Data Store Read23/1 -> Logical Operator11/4
  [CANvalid_bus] -> Bus Selector19/1
  Bus Selector19/1 -> Logical Operator10/1  "<VINP_CANMsgValidVCU1_flg>"
  Bus Selector19/2 -> Logical Operator10/2  "<VINP_CANMsgUpdatedVCU1_flg>"
  Bus Selector19/3 -> Logical Operator10/3  "<VINP_CANMsgCheckedVCU1_flg>"
  Logical Operator10/1 -> AckCurVehDec/3(msg_valid)
  Bus Selector24/1 -> DeceContManag/1(signal_in)  "<VHAL_DeceContManag_flg>"
  [BHAL_outputs] -> Bus Selector24/1
  DeceContManag/1(signal_out) -> inputs_bus2/14  "VINP_DeceContManag_flg_InHAL"
  DeceContManag/2(signal_valid_out) -> inputs_bus2/15  "VINP_DeceContManagValid_flg_InHAL"
  Data Store Read29/1 -> Logical Operator16/1
  Logical Operator16/1 -> DeceContManag/3(Active_fault_flg)
  Data Store Read30/1 -> Logical Operator16/2
  Data Store Read28/1 -> Logical Operator16/3
  [CANvalid_bus] -> Bus Selector23/1
  Bus Selector23/1 -> Logical Operator15/1  "<VINP_CANMsgValidVCU1_flg>"
  Bus Selector23/2 -> Logical Operator15/2  "<VINP_CANMsgUpdatedVCU1_flg>"
  Bus Selector23/3 -> Logical Operator15/3  "<VINP_CANMsgCheckedVCU1_flg>"
  Logical Operator15/1 -> DeceContManag/2(msg_valid)
  Bus Selector28/1 -> ECMExtnTqAvailable/1(signal_in)  "<VHAL_ECMExtnTqAvailable_flg>"
  [BHAL_outputs] -> Bus Selector28/1
  ECMExtnTqAvailable/1(signal_out) -> inputs_bus2/19  "VINP_ECMExtnTqAvailable_flg_InHAL"
  ECMExtnTqAvailable/2(signal_valid_out) -> inputs_bus2/20  "VINP_ECMExtnTqAvailableValid_flg_InHAL"
  Data Store Read35/1 -> Logical Operator21/1
  Logical Operator21/1 -> ECMExtnTqAvailable/3(Active_fault_flg)
  Data Store Read36/1 -> Logical Operator21/2
  Data Store Read34/1 -> Logical Operator21/3
  [CANvalid_bus] -> Bus Selector27/1
  Bus Selector27/1 -> Logical Operator20/1  "<VINP_CANMsgValidVCU1_flg>"
  Bus Selector27/2 -> Logical Operator20/2  "<VINP_CANMsgUpdatedVCU1_flg>"
  Bus Selector27/3 -> Logical Operator20/3  "<VINP_CANMsgCheckedVCU1_flg>"
  Logical Operator20/1 -> ECMExtnTqAvailable/2(msg_valid)
  Bus Selector30/1 -> ECMTqSource/1(signal_in)  "<VHAL_ECMTqSource_enum>"
  [BHAL_outputs] -> Bus Selector30/1
  ECMTqSource/1(signal_out) -> inputs_bus2/21  "VINP_ECMTqSource_enum_InHAL"
  ECMTqSource/2(signal_valid_out) -> inputs_bus2/22  "VINP_ECMTqSourceValid_flg_InHAL"
  Data Store Read38/1 -> Logical Operator23/1
  Logical Operator23/1 -> ECMTqSource/3(Active_fault_flg)
  Data Store Read39/1 -> Logical Operator23/2
  Data Store Read37/1 -> Logical Operator23/3
  [CANvalid_bus] -> Bus Selector29/1
  Bus Selector29/1 -> Logical Operator22/1  "<VINP_CANMsgValidVCU1_flg>"
  Bus Selector29/2 -> Logical Operator22/2  "<VINP_CANMsgUpdatedVCU1_flg>"
  Bus Selector29/3 -> Logical Operator22/3  "<VINP_CANMsgCheckedVCU1_flg>"
  Logical Operator22/1 -> ECMTqSource/2(msg_valid)
  Bus Selector32/1 -> ESCBrakeStatus/1(signal_in)  "<VHAL_ESCBrakeStatus_enum>"
  [BHAL_outputs] -> Bus Selector32/1
  ESCBrakeStatus/1(signal_out) -> inputs_bus2/23  "VINP_ESCBrakeStatus_enum_InHAL"
  ESCBrakeStatus/2(signal_valid_out) -> inputs_bus2/24  "VINP_ESCBrakeStatusValid_flg_InHAL"
  Data Store Read41/1 -> Logical Operator25/1
  Logical Operator25/1 -> ESCBrakeStatus/3(Active_fault_flg)
  Data Store Read42/1 -> Logical Operator25/2
  Data Store Read40/1 -> Logical Operator25/3
  [CANvalid_bus] -> Bus Selector31/1
  Bus Selector31/1 -> Logical Operator24/1  "<VINP_CANMsgValidVCU1_flg>"
  Bus Selector31/2 -> Logical Operator24/2  "<VINP_CANMsgUpdatedVCU1_flg>"
  Bus Selector31/3 -> Logical Operator24/3  "<VINP_CANMsgCheckedVCU1_flg>"
  Logical Operator24/1 -> ESCBrakeStatus/2(msg_valid)
  Bus Selector34/1 -> ESCExtnBrkAvailable/1(signal_in)  "<VHAL_ESCExtnBrkAvailable_flg>"
  [BHAL_outputs] -> Bus Selector34/1
  ESCExtnBrkAvailable/1(signal_out) -> inputs_bus2/25  "VINP_ESCExtnBrkAvailable_flg_InHAL"
  ESCExtnBrkAvailable/2(signal_valid_out) -> inputs_bus2/26  "VINP_ESCExtnBrkAvailableValid_flg_InHAL"
  Data Store Read44/1 -> Logical Operator27/1
  Logical Operator27/1 -> ESCExtnBrkAvailable/3(Active_fault_flg)
  Data Store Read45/1 -> Logical Operator27/2
  Data Store Read43/1 -> Logical Operator27/3
  [CANvalid_bus] -> Bus Selector33/1
  Bus Selector33/1 -> Logical Operator26/1  "<VINP_CANMsgValidVCU1_flg>"
  Bus Selector33/2 -> Logical Operator26/2  "<VINP_CANMsgUpdatedVCU1_flg>"
  Bus Selector33/3 -> Logical Operator26/3  "<VINP_CANMsgCheckedVCU1_flg>"
  Logical Operator26/1 -> ESCExtnBrkAvailable/2(msg_valid)
  [BHAL_outputs] -> Bus Selector36/1
  Bus Selector36/1 -> ESCBattVolt/1(signal_in)  "<VHAL_ESCBattVolt_V>"
  Bus Selector36/2 -> Logical Operator28/1  "<VHAL_ESCBattVoltInvalid_flg>"
  Logical Operator28/1 -> ESCBattVolt/2(signal_valid_in)
  Data Store Read49/1 -> Logical Operator30/1
  Data Store Read48/1 -> Logical Operator30/2
  ESCBattVolt/1(signal_out) -> inputs_bus2/27  "VINP_ESCBattVolt_V_InHAL"
  ESCBattVolt/2(signal_valid_out) -> inputs_bus2/28  "VINP_ESCBattVoltValid_flg_InHAL"
  ESCBattVolt/3(raw_signal_valid_out) -> inputs_bus2/29  "VINP_ESCBattVoltSigOk_flg_InHAL"
  Logical Operator30/1 -> ESCBattVolt/4(Active_fault_flg)
  Data Store Read46/1 -> Logical Operator30/3
  Data Store Read47/1 -> Logical Operator30/4
  [CANvalid_bus] -> Bus Selector35/1
  Bus Selector35/1 -> Logical Operator29/1  "<VINP_CANMsgValidVCU1_flg>"
  Bus Selector35/2 -> Logical Operator29/2  "<VINP_CANMsgUpdatedVCU1_flg>"
  Bus Selector35/3 -> Logical Operator29/3  "<VINP_CANMsgCheckedVCU1_flg>"
  Logical Operator29/1 -> ESCBattVolt/3(msg_valid)
  [BHAL_outputs] -> Bus Selector38/1
  Bus Selector38/1 -> HWAccelPedalPosn/1(signal_in)  "<VHAL_HWAccelPedalPosn_pct>"
  Bus Selector38/2 -> Logical Operator31/1  "<VHAL_HWAccelPedalPosnInvalid_flg>"
  Logical Operator31/1 -> HWAccelPedalPosn/2(signal_valid_in)
  Data Store Read53/1 -> Logical Operator33/1
  Data Store Read52/1 -> Logical Operator33/2
  HWAccelPedalPosn/1(signal_out) -> inputs_bus2/30  "VINP_HWAccelPedalPosn_pct_InHAL"
  HWAccelPedalPosn/2(signal_valid_out) -> inputs_bus2/31  "VINP_HWAccelPedalPosnValid_flg_InHAL"
  HWAccelPedalPosn/3(raw_signal_valid_out) -> inputs_bus2/32  "VINP_HWAccelPedalPosnSigOk_flg_InHAL"
  Logical Operator33/1 -> HWAccelPedalPosn/4(Active_fault_flg)
  Data Store Read50/1 -> Logical Operator33/3
  Data Store Read51/1 -> Logical Operator33/4
  [CANvalid_bus] -> Bus Selector37/1
  Bus Selector37/1 -> Logical Operator32/1  "<VINP_CANMsgValidVCU2_flg>"
  Bus Selector37/2 -> Logical Operator32/2  "<VINP_CANMsgUpdatedVCU2_flg>"
  Bus Selector37/3 -> Logical Operator32/3  "<VINP_CANMsgCheckedVCU2_flg>"
  Logical Operator32/1 -> HWAccelPedalPosn/3(msg_valid)
  Bus Selector44/1 -> HCUMILReq/2(msg_valid)  "<VINP_CANMsgValidHCU4_flg>"
  [CANvalid_bus] -> Bus Selector44/1
  Bus Selector43/1 -> HCUMILReq/1(signal_in)  "<VHAL_HCUMILReq_flg>"
  [BHAL_outputs] -> Bus Selector43/1
  HCUMILReq/1(signal_out) -> inputs_bus2/42  "VINP_HCUMILReq_flg_InHAL"
  HCUMILReq/2(signal_valid_out) -> inputs_bus2/43  "VINP_HCUMILReqValid_flg_InHAL"
  Data Store Read54/1 -> HCUMILReq/3(Active_fault_flg)
  [BHAL_outputs] -> Bus Selector46/1
  Bus Selector46/1 -> CurDriverTqReq/1(signal_in)  "<VHAL_CurDriverTqReq_Nm>"
  Bus Selector46/2 -> Logical Operator36/1  "<VHAL_CurDriverTqReqInvalid_flg>"
  Logical Operator36/1 -> CurDriverTqReq/2(signal_valid_in)
  Data Store Read60/1 -> Logical Operator38/1
  Data Store Read59/1 -> Logical Operator38/2
  CurDriverTqReq/1(signal_out) -> inputs_bus2/33  "VINP_CurDriverTqReq_Nm_InHAL"
  CurDriverTqReq/2(signal_valid_out) -> inputs_bus2/34  "VINP_CurDriverTqReqValid_flg_InHAL"
  CurDriverTqReq/3(raw_signal_valid_out) -> inputs_bus2/35  "VINP_CurDriverTqReqSigOk_flg_InHAL"
  Logical Operator38/1 -> CurDriverTqReq/4(Active_fault_flg)
  Data Store Read57/1 -> Logical Operator38/3
  Data Store Read58/1 -> Logical Operator38/4
  [CANvalid_bus] -> Bus Selector45/1
  Bus Selector45/1 -> Logical Operator37/1  "<VINP_CANMsgValidVCU2_flg>"
  Bus Selector45/2 -> Logical Operator37/2  "<VINP_CANMsgUpdatedVCU2_flg>"
  Bus Selector45/3 -> Logical Operator37/3  "<VINP_CANMsgCheckedVCU2_flg>"
  Logical Operator37/1 -> CurDriverTqReq/3(msg_valid)
  Bus Selector47/1 -> MSAOnVCU/2(msg_valid)  "<VINP_CANMsgValidHMI2_flg>"
  [CANvalid_bus] -> Bus Selector47/1
  Bus Selector48/1 -> MSAOnVCU/1(signal_in)  "<VHAL_MSAOnVCU_flg>"
  [BHAL_outputs] -> Bus Selector48/1
  MSAOnVCU/1(signal_out) -> inputs_bus2/56  "VINP_MSAOnVCU_flg_InHAL"
  MSAOnVCU/2(signal_valid_out) -> inputs_bus2/57  "VINP_MSAOnVCUValid_flg_InHAL"
  Data Store Read61/1 -> MSAOnVCU/3(Active_fault_flg)
  Bus Selector22/1 -> DriverBrakPedalOn/1(signal_in)  "<VHAL_DriverBrakPedalOn_flg>"
  [BHAL_outputs] -> Bus Selector22/1
  Logical Operator12/1 -> DriverBrakPedalOn/2(signal_valid_in)
  DriverBrakPedalOn/1(signal_out) -> inputs_bus2/16  "VINP_DriverBrakPedalOn_flg_InHAL"
  DriverBrakPedalOn/2(signal_valid_out) -> inputs_bus2/17  "VINP_DriverBrakPedalOnValid_flg_InHAL"
  Bus Selector22/2 -> Logical Operator12/1  "<VHAL_DriverBrakPedalOnInvalid_flg>"
  Data Store Read32/1 -> Logical Operator39/2
  Data Store Read33/1 -> Logical Operator39/3
  Logical Operator39/1 -> DriverBrakPedalOn/4(Active_fault_flg)
  Data Store Read62/1 -> Logical Operator39/1
  DriverBrakPedalOn/3(raw_signal_valid_out) -> inputs_bus2/18  "VINP_DriverBrakPedalOnSigOk_flg_InHAL"
  Logical Operator13/1 -> DriverBrakPedalOn/3(msg_valid)
  Data Store Read31/1 -> Logical Operator39/4
  [CANvalid_bus] -> Bus Selector25/1
  Bus Selector25/1 -> Logical Operator13/1  "<VINP_CANMsgValidVCU1_flg>"
  Bus Selector25/2 -> Logical Operator13/2  "<VINP_CANMsgUpdatedVCU1_flg>"
  Bus Selector25/3 -> Logical Operator13/3  "<VINP_CANMsgCheckedVCU1_flg>"
  Bus Selector21/1 -> Data Type Conversion/1  "<VHAL_TCMGearPosnReqAllow_enum>"
  [BHAL_outputs] -> Bus Selector21/1
  Data Type Conversion/1 -> inputs_bus2/36  "VINP_TCMGearPosnReqAllow_enum_InHAL"
  Constant/1 -> inputs_bus2/37  "VINP_TCMGearPosnReqAllowValid_flg_InHAL"
  Data Store Read27/1 -> Logical Operator17/3
  Data Store Read25/1 -> Logical Operator17/2
  Data Store Read55/1 -> Logical Operator17/1
  Logical Operator17/1 -> Terminator1/1
  [CANvalid_bus] -> Bus Selector26/1
  Bus Selector26/1 -> Logical Operator18/1  "<VINP_CANMsgValidVCU2_flg>"
  Bus Selector26/2 -> Logical Operator18/2  "<VINP_CANMsgUpdatedVCU2_flg>"
  Bus Selector26/3 -> Logical Operator18/3  "<VINP_CANMsgCheckedVCU2_flg>"
  Logical Operator18/1 -> Terminator2/1
  Bus Selector41/1 -> Data Type Conversion1/1  "<VHAL_MSAStatus_enum>"
  [BHAL_outputs] -> Bus Selector41/1
  Data Type Conversion1/1 -> inputs_bus2/38  "VINP_MSAStatus_enum_InHAL"
  Constant1/1 -> inputs_bus2/39  "VINP_MSAStatusValid_flg_InHAL"
  Data Store Read56/1 -> Logical Operator34/3
  Data Store Read24/1 -> Logical Operator34/2
  Data Store Read63/1 -> Logical Operator34/1
  Logical Operator34/1 -> Terminator3/1
  [CANvalid_bus] -> Bus Selector42/1
  Bus Selector42/1 -> Logical Operator35/1  "<VINP_CANMsgValidVCU2_flg>"
  Bus Selector42/2 -> Logical Operator35/2  "<VINP_CANMsgUpdatedVCU2_flg>"
  Bus Selector42/3 -> Logical Operator35/3  "<VINP_CANMsgCheckedVCU2_flg>"
  Logical Operator35/1 -> Terminator4/1
  Bus Selector49/1 -> Data Type Conversion2/1  "<VHAL_HybridLimpHome_flg>"
  [BHAL_outputs] -> Bus Selector49/1
  Data Type Conversion2/1 -> inputs_bus2/40  "VINP_HybridLimpHome_flg_InHAL"
  Constant2/1 -> inputs_bus2/41  "VINP_HybridLimpHomeValid_flg_InHAL"
  Data Store Read64/1 -> Logical Operator40/2
  Data Store Read66/1 -> Logical Operator40/1
  Logical Operator40/1 -> Terminator5/1
  [CANvalid_bus] -> Bus Selector50/1
  Bus Selector50/1 -> Logical Operator41/1  "<VINP_CANMsgValidHCU3_flg>"
  Bus Selector50/2 -> Logical Operator41/2  "<VINP_CANMsgUpdatedHCU3_flg>"
  Logical Operator41/1 -> Terminator6/1
